simulink model "ClimaAdjmtOfTotFlowMgr"  (R2022b) (continued)
== system ClimaAdjmtOfTotFlowMgr == (continued)
  AirTFromHeatgEstimdForCCM_AirTFromHeatgEstimd/1 -> ClimaAdjmtOfTotFlowMgr_Runnable_sys/61(AirTFromHeatgEstimdForCCM_AirTFromHeatgEstimd_read)
  DrvrPfmncAlrmReq_DrvrPfmncAlrmReq/1 -> ClimaAdjmtOfTotFlowMgr_Runnable_sys/62(DrvrPfmncAlrmReq_DrvrPfmncAlrmReq_read)
  DrvrPfmncAlrmReqFromDHU_DrvrPfmncAlrmReqFromDHU/1 -> ClimaAdjmtOfTotFlowMgr_Runnable_sys/63(DrvrPfmncAlrmReqFromDHU_DrvrPfmncAlrmReqFromDHU_read)
  ClimaCfgForAirMtrlSnsrPrsnt1_ClimaCfgForAirMtrlSnsrPrsnt1/1 -> ClimaAdjmtOfTotFlowMgr_Runnable_sys/64(ClimaCfgForAirMtrlSnsrPrsnt1_ClimaCfgForAirMtrlSnsrPrsnt1_read)
  ClimaCfgForErev1_ClimaCfgForErev1/1 -> ClimaAdjmtOfTotFlowMgr_Runnable_sys/65(ClimaCfgForErev1_ClimaCfgForErev1_read)
  ClimaCfgReqVersionReplace_ClimaCfgReqVersionReplace/1 -> ClimaAdjmtOfTotFlowMgr_Runnable_sys/66(ClimaCfgReqVersionReplace_ClimaCfgReqVersionReplace_read)
  ClimaStStrtUpRe_ClimaStStrtUpRe/1 -> ClimaAdjmtOfTotFlowMgr_Runnable_sys/67(ClimaStStrtUpRe_ClimaStStrtUpRe_read)
  FlowRednForEngStby_FlowRednForEngStby/1 -> ClimaAdjmtOfTotFlowMgr_Runnable_sys/68(FlowRednForEngStby_FlowRednForEngStby_read)
  HmiCmptmtTSpForClima_HmiCmptmtTSpForClima/1 -> ClimaAdjmtOfTotFlowMgr_Runnable_sys/69(HmiCmptmtTSpForClima_HmiCmptmtTSpForClima_read)
  HvacAirTForRowFirstAtFlrLeRaw_HvacAirTForRowFirstAtFlrLeRaw/1 -> ClimaAdjmtOfTotFlowMgr_Runnable_sys/70(HvacAirTForRowFirstAtFlrLeRaw_HvacAirTForRowFirstAtFlrLeRaw_read)
  PrkgClimaOnReq_PrkgClimaOnReq/1 -> ClimaAdjmtOfTotFlowMgr_Runnable_sys/71(PrkgClimaOnReq_PrkgClimaOnReq_read)
  PrkgClimaOnReqRe_PrkgClimaOnReqRe/1 -> ClimaAdjmtOfTotFlowMgr_Runnable_sys/72(PrkgClimaOnReqRe_PrkgClimaOnReqRe_read)
  TModCtrlAtRowSec_TModCtrlAtRowSec/1 -> ClimaAdjmtOfTotFlowMgr_Runnable_sys/73(TModCtrlAtRowSec_TModCtrlAtRowSec_read)
  TModCtrlAtRowSec_TModCtrlAtRowSecLe/1 -> ClimaAdjmtOfTotFlowMgr_Runnable_sys/74(TModCtrlAtRowSec_TModCtrlAtRowSecLe_read)
  TModCtrlAtRowSec_TModCtrlAtRowSecRi/1 -> ClimaAdjmtOfTotFlowMgr_Runnable_sys/75(TModCtrlAtRowSec_TModCtrlAtRowSecRi_read)
  ClimaAdjmtOfTotFlowMgr_Runnable_sys/1(FlowDecDurgSpdIdleActvFlg_FlowDecDurgSpdIdleActvFlg_write) -> FlowDecDurgSpdIdleActvFlg_FlowDecDurgSpdIdleActvFlg/1
  ClimaAdjmtOfTotFlowMgr_Runnable_sys/2(FlowDecDurgSpdIdleActvFlgRe_FlowDecDurgSpdIdleActvFlgRe_write) -> FlowDecDurgSpdIdleActvFlgRe_FlowDecDurgSpdIdleActvFlgRe/1
  ClimaAdjmtOfTotFlowMgr_Runnable_sys/3(AirFlowLimForAirTLoActv_AirFlowLimForAirTLoActv_write) -> AirFlowLimForAirTLoActv_AirFlowLimForAirTLoActv/1
  ClimaAdjmtOfTotFlowMgr_Runnable_sys/4(ChgOfAirFlowForEngStb_ChgOfAirFlowForEngStb_write) -> ChgOfAirFlowForEngStb_ChgOfAirFlowForEngStb/1
  ClimaAdjmtOfTotFlowMgr_Runnable_sys/5(ChgOfFanLvlAut_ChgOfFanLvlAut_write) -> ChgOfFanLvlAut_ChgOfFanLvlAut/1
  ClimaAdjmtOfTotFlowMgr_Runnable_sys/6(ClimaAutCtrldFlowPredForDefrstAtStaby_ClimaAutCtrldFlowPredForDefrstAtStaby_write) -> ClimaAutCtrldFlowPredForDefrstAtStaby_ClimaAutCtrldFlowPredForDefrstAtStaby/1
  ClimaAdjmtOfTotFlowMgr_Runnable_sys/7(ClimaAutCtrldFlowPredForDefrstNoLim_ClimaAutCtrldFlowPredForDefrstNoLim_write) -> ClimaAutCtrldFlowPredForDefrstNoLim_ClimaAutCtrldFlowPredForDefrstNoLim/1
  ClimaAdjmtOfTotFlowMgr_Runnable_sys/8(ClimaAutCtrldFlowPredForRowFirstAtStaby_ClimaAutCtrldFlowPredForRowFirstAtStaby_write) -> ClimaAutCtrldFlowPredForRowFirstAtStaby_ClimaAutCtrldFlowPredForRowFirstAtStaby/1
  ClimaAdjmtOfTotFlowMgr_Runnable_sys/9(ClimaAutCtrldFlowPredForRowFirstNoLim_ClimaAutCtrldFlowPredForRowFirstNoLim_write) -> ClimaAutCtrldFlowPredForRowFirstNoLim_ClimaAutCtrldFlowPredForRowFirstNoLim/1
  ClimaAdjmtOfTotFlowMgr_Runnable_sys/10(ClimaAutCtrldFlowPredForRowSecAtStaby_ClimaAutCtrldFlowPredForRowSecAtStaby_write) -> ClimaAutCtrldFlowPredForRowSecAtStaby_ClimaAutCtrldFlowPredForRowSecAtStaby/1
  ClimaAdjmtOfTotFlowMgr_Runnable_sys/11(ClimaAutCtrldFlowPredForRowSecNoLim_ClimaAutCtrldFlowPredForRowSecNoLim_write) -> ClimaAutCtrldFlowPredForRowSecNoLim_ClimaAutCtrldFlowPredForRowSecNoLim/1
  ClimaAdjmtOfTotFlowMgr_Runnable_sys/12(ClimaAutCtrldFlowReqForDefrst_ClimaAutCtrldFlowReqForDefrst_write) -> ClimaAutCtrldFlowReqForDefrst_ClimaAutCtrldFlowReqForDefrst/1
  ClimaAdjmtOfTotFlowMgr_Runnable_sys/13(ClimaAutCtrldFlowReqForDefrst_ClimaAutCtrldFlowReqForDefrstLe_write) -> ClimaAutCtrldFlowReqForDefrst_ClimaAutCtrldFlowReqForDefrstLe/1
  ClimaAdjmtOfTotFlowMgr_Runnable_sys/14(ClimaAutCtrldFlowReqForDefrst_ClimaAutCtrldFlowReqForDefrstRi_write) -> ClimaAutCtrldFlowReqForDefrst_ClimaAutCtrldFlowReqForDefrstRi/1
  ClimaAdjmtOfTotFlowMgr_Runnable_sys/15(ClimaAutCtrldFlowReqForDefrstNoLim_ClimaAutCtrldFlowReqForDefrstNoLim_write) -> ClimaAutCtrldFlowReqForDefrstNoLim_ClimaAutCtrldFlowReqForDefrstNoLim/1
  ClimaAdjmtOfTotFlowMgr_Runnable_sys/16(ClimaAutCtrldTotFlowReqForRowFirst_ClimaAutCtrldTotFlowReqForRowFirst_write) -> ClimaAutCtrldTotFlowReqForRowFirst_ClimaAutCtrldTotFlowReqForRowFirst/1
  ClimaAdjmtOfTotFlowMgr_Runnable_sys/17(ClimaAutCtrldTotFlowReqForRowFirst_ClimaAutCtrldTotFlowReqForRowFirstLe_write) -> ClimaAutCtrldTotFlowReqForRowFirst_ClimaAutCtrldTotFlowReqForRowFirstLe/1
  ClimaAdjmtOfTotFlowMgr_Runnable_sys/18(ClimaAutCtrldTotFlowReqForRowFirst_ClimaAutCtrldTotFlowReqForRowFirstRi_write) -> ClimaAutCtrldTotFlowReqForRowFirst_ClimaAutCtrldTotFlowReqForRowFirstRi/1
  ClimaAdjmtOfTotFlowMgr_Runnable_sys/19(ClimaAutCtrldTotFlowReqForRowFirstNoLim_ClimaAutCtrldTotFlowReqForRowFirstNoLim_write) -> ClimaAutCtrldTotFlowReqForRowFirstNoLim_ClimaAutCtrldTotFlowReqForRowFirstNoLim/1
  ClimaAdjmtOfTotFlowMgr_Runnable_sys/20(ClimaAutCtrldTotFlowReqForRowSec_ClimaAutCtrldTotFlowReqForRowSec_write) -> ClimaAutCtrldTotFlowReqForRowSec_ClimaAutCtrldTotFlowReqForRowSec/1
  ClimaAdjmtOfTotFlowMgr_Runnable_sys/21(ClimaAutCtrldTotFlowReqForRowSec_ClimaAutCtrldTotFlowReqForRowSecLe_write) -> ClimaAutCtrldTotFlowReqForRowSec_ClimaAutCtrldTotFlowReqForRowSecLe/1
  ClimaAdjmtOfTotFlowMgr_Runnable_sys/22(ClimaAutCtrldTotFlowReqForRowSec_ClimaAutCtrldTotFlowReqForRowSecRi_write) -> ClimaAutCtrldTotFlowReqForRowSec_ClimaAutCtrldTotFlowReqForRowSecRi/1
  ClimaAdjmtOfTotFlowMgr_Runnable_sys/23(ClimaAutCtrldTotFlowReqForRowSecNoLim_ClimaAutCtrldTotFlowReqForRowSecNoLim_write) -> ClimaAutCtrldTotFlowReqForRowSecNoLim_ClimaAutCtrldTotFlowReqForRowSecNoLim/1
  ClimaAdjmtOfTotFlowMgr_Runnable_sys/24(FanLvlMaxActv_FanLvlMaxActv_write) -> FanLvlMaxActv_FanLvlMaxActv/1
  ClimaAdjmtOfTotFlowMgr_Runnable/1 -> ClimaAdjmtOfTotFlowMgr_Runnable_sys/Trigger

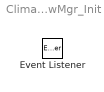
== system ClimaAdjmtOfTotFlowMgr/ClimaAdjmtOfTotFlowMgr_Init ==
  Event Listener : EventListener  EventName=reset

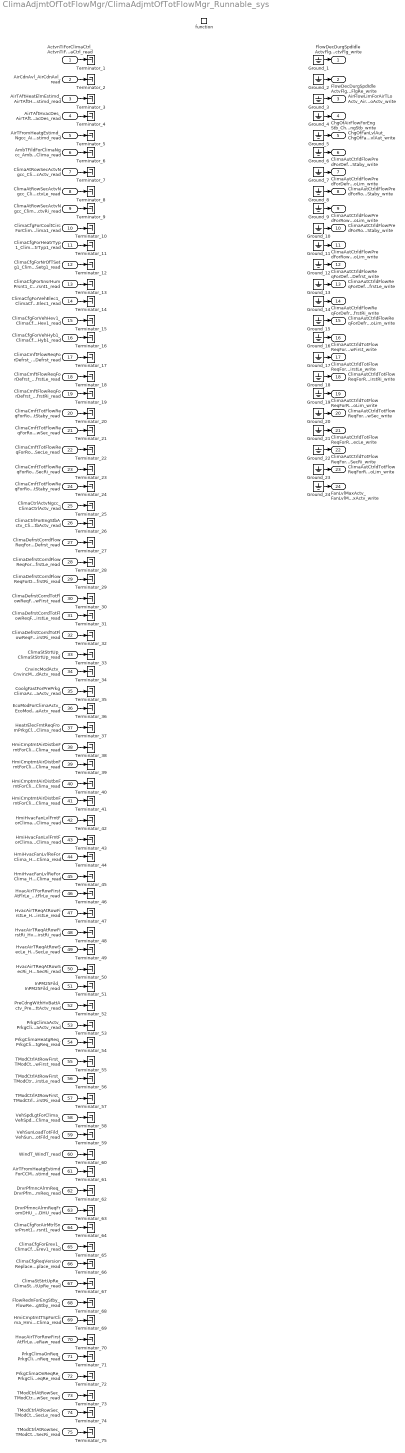
== system ClimaAdjmtOfTotFlowMgr/ClimaAdjmtOfTotFlowMgr_Runnable_sys ==
  ActvnTiForClimaCtrl_ActvnTiForClimaCtrl_read : Inport
  AirCdnAvl_AirCdnAvl_read : Inport  Port=2
  AirTAftHeatElmEstimd_AirTAftHeatElmEstimd_read : Inport  Port=3
  AirTAftHvacDes_AirTAftHvacDes_read : Inport  Port=4
  AirTFromHeatgEstimd_Ngcc_AirTFromHeatgEstimd_read : Inport  Port=5
  AmbTFildForClimaNgcc_AmbTFildForClima_read : Inport  Port=6
  ClimaAtRowSecActvNgcc_ClimaAtRowSecActv_read : Inport  Port=7
  ClimaAtRowSecActvNgcc_ClimaAtRowSecActvLe_read : Inport  Port=8
  ClimaAtRowSecActvNgcc_ClimaAtRowSecActvRi_read : Inport  Port=9
  ClimaCfgForCooltCircForClima1_ClimaCfgForCooltCircForClima1_read : Inport  Port=10
  ClimaCfgForHeatrTyp1_ClimaCfgForHeatrTyp1_read : Inport  Port=11
  ClimaCfgForNrOfTSetg1_ClimaCfgForNrOfTSetg1_read : Inport  Port=12
  ClimaCfgForSnsrHumPrsnt1_ClimaCfgForSnsrHumPrsnt1_read : Inport  Port=13
  ClimaCfgForVehElec1_ClimaCfgForVehElec1_read : Inport  Port=14
  ClimaCfgForVehHev1_ClimaCfgForVehHev1_read : Inport  Port=15
  ClimaCfgForVehHyb1_ClimaCfgForVehHyb1_read : Inport  Port=16
  ClimaCmftFlowReqForDefrst_ClimaCmftFlowReqForDefrst_read : Inport  Port=17
  ClimaCmftFlowReqForDefrst_ClimaCmftFlowReqForDefrstLe_read : Inport  Port=18
  ClimaCmftFlowReqForDefrst_ClimaCmftFlowReqForDefrstRi_read : Inport  Port=19
  ClimaCmftTotFlowReqForRowFirstAtStaby_ClimaCmftTotFlowReqForRowFirstAtStaby_read : Inport  Port=20
  ClimaCmftTotFlowReqForRowSec_ClimaCmftTotFlowReqForRowSec_read : Inport  Port=21
  ClimaCmftTotFlowReqForRowSec_ClimaCmftTotFlowReqForRowSecLe_read : Inport  Port=22
  ClimaCmftTotFlowReqForRowSec_ClimaCmftTotFlowReqForRowSecRi_read : Inport  Port=23
  ClimaCmftTotFlowReqForRowSecAtStaby_ClimaCmftTotFlowReqForRowSecAtStaby_read : Inport  Port=24
  ClimaCtrlActvNgcc_ClimaCtrlActv_read : Inport  Port=25
  ClimaCtrlForEngStbActv_ClimaCtrlForEngStbActv_read : Inport  Port=26
  ClimaDefrstCorrdFlowReqForDefrst_ClimaDefrstCorrdFlowReqForDefrst_read : Inport  Port=27
  ClimaDefrstCorrdFlowReqForDefrst_ClimaDefrstCorrdFlowReqForDefrstLe_read : Inport  Port=28
  ClimaDefrstCorrdFlowReqForDefrst_ClimaDefrstCorrdFlowReqForDefrstRi_read : Inport  Port=29
  ClimaDefrstCorrdTotFlowReqForRowFirst_ClimaDefrstCorrdTotFlowReqForRowFirst_read : Inport  Port=30
  ClimaDefrstCorrdTotFlowReqForRowFirst_ClimaDefrstCorrdTotFlowReqForRowFirstLe_read : Inport  Port=31
  ClimaDefrstCorrdTotFlowReqForRowFirst_ClimaDefrstCorrdTotFlowReqForRowFirstRi_read : Inport  Port=32
  ClimaStStrtUp_ClimaStStrtUp_read : Inport  Port=33
  CnvincModActv_CnvincModActv_read : Inport  Port=34
  CoolgFastForPrePrkgClimaActv_CoolgFastForPrePrkgClimaActv_read : Inport  Port=35
  EcoModForClimaActv_EcoModForClimaActv_read : Inport  Port=36
  HeatrElecFrntReqFromPrkgClima_HeatrElecFrntReqFromPrkgClima_read : Inport  Port=37
  HmiCmptmtAirDistbnFrntForClima_HmiCmptmtAirDistbnFrntForClima_read : Inport  Port=38
  HmiCmptmtAirDistbnFrntForClima_HmiCmptmtAirDistbnFrntRiForClima_read : Inport  Port=39
  HmiCmptmtAirDistbnFrntForClima_HmiCmptmtAirDistbnReForClima_read : Inport  Port=40
  HmiCmptmtAirDistbnFrntForClima_HmiCmptmtAirDistbnReRiForClima_read : Inport  Port=41
  HmiHvacFanLvlFrntForClima_HmiHvacFanLvlFrntForClima_read : Inport  Port=42
  HmiHvacFanLvlFrntForClima_HmiHvacFanLvlFrntRiForClima_read : Inport  Port=43
  HmiHvacFanLvlReForClima_HmiHvacFanLvlReForClima_read : Inport  Port=44
  HmiHvacFanLvlReForClima_HmiHvacFanLvlReRiForClima_read : Inport  Port=45
  HvacAirTForRowFirstAtFlrLe_HvacAirTForRowFirstAtFlrLe_HvacAirTForRowFirstAtFlrLe_read : Inport  Port=46
  HvacAirTReqAtRowFirstLe_HvacAirTReqAtRowFirstLe_read : Inport  Port=47
  HvacAirTReqAtRowFirstRi_HvacAirTReqAtRowFirstRi_read : Inport  Port=48
  HvacAirTReqAtRowSecLe_HvacAirTReqAtRowSecLe_read : Inport  Port=49
  HvacAirTReqAtRowSecRi_HvacAirTReqAtRowSecRi_read : Inport  Port=50
  InPM25Fild_InPM25Fild_read : Inport  Port=51
  PreCdngWithHvBattActv_PreCdngWithHvBattActv_read : Inport  Port=52
  PrkgClimaActv_PrkgClimaActv_read : Inport  Port=53
  PrkgClimaHeatgReq_PrkgClimaHeatgReq_read : Inport  Port=54
  TModCtrlAtRowFirst_TModCtrlAtRowFirst_read : Inport  Port=55
  TModCtrlAtRowFirst_TModCtrlAtRowFirstLe_read : Inport  Port=56
  TModCtrlAtRowFirst_TModCtrlAtRowFirstRi_read : Inport  Port=57
  VehSpdLgtForClima_VehSpdLgtForClima_read : Inport  Port=58
  VehSunLoadTotFild_VehSunLoadTotFild_read : Inport  Port=59
  WindT_WindT_read : Inport  Port=60
  AirTFromHeatgEstimdForCCM_AirTFromHeatgEstimd_read : Inport  Port=61
  DrvrPfmncAlrmReq_DrvrPfmncAlrmReq_read : Inport  Port=62
  DrvrPfmncAlrmReqFromDHU_DrvrPfmncAlrmReqFromDHU_read : Inport  Port=63
  ClimaCfgForAirMtrlSnsrPrsnt1_ClimaCfgForAirMtrlSnsrPrsnt1_read : Inport  Port=64
  ClimaCfgForErev1_ClimaCfgForErev1_read : Inport  Port=65
  ClimaCfgReqVersionReplace_ClimaCfgReqVersionReplace_read : Inport  Port=66
  ClimaStStrtUpRe_ClimaStStrtUpRe_read : Inport  Port=67
  FlowRednForEngStby_FlowRednForEngStby_read : Inport  Port=68
  HmiCmptmtTSpForClima_HmiCmptmtTSpForClima_read : Inport  Port=69
  HvacAirTForRowFirstAtFlrLeRaw_HvacAirTForRowFirstAtFlrLeRaw_read : Inport  Port=70
  PrkgClimaOnReq_PrkgClimaOnReq_read : Inport  Port=71
  PrkgClimaOnReqRe_PrkgClimaOnReqRe_read : Inport  Port=72
  TModCtrlAtRowSec_TModCtrlAtRowSec_read : Inport  Port=73
  TModCtrlAtRowSec_TModCtrlAtRowSecLe_read : Inport  Port=74
  TModCtrlAtRowSec_TModCtrlAtRowSecRi_read : Inport  Port=75
  function : TriggerPort  TriggerType=function-call  VariantControl=(inherit)  SampleTimeType=periodic  SampleTime=-1
  Ground_{1..24} (24 alike) : Ground
  Terminator_{1..75} (75 alike) : Terminator
  FlowDecDurgSpdIdleActvFlg_FlowDecDurgSpdIdleActvFlg_write : Outport
      settings: EnsureOutportIsVirtual=on
  FlowDecDurgSpdIdleActvFlgRe_FlowDecDurgSpdIdleActvFlgRe_write : Outport  Port=2
      settings: EnsureOutportIsVirtual=on
  AirFlowLimForAirTLoActv_AirFlowLimForAirTLoActv_write : Outport  Port=3
      settings: EnsureOutportIsVirtual=on
  ChgOfAirFlowForEngStb_ChgOfAirFlowForEngStb_write : Outport  Port=4
      settings: EnsureOutportIsVirtual=on
  ChgOfFanLvlAut_ChgOfFanLvlAut_write : Outport  Port=5
      settings: EnsureOutportIsVirtual=on
  ClimaAutCtrldFlowPredForDefrstAtStaby_ClimaAutCtrldFlowPredForDefrstAtStaby_write : Outport  Port=6
      settings: EnsureOutportIsVirtual=on
  ClimaAutCtrldFlowPredForDefrstNoLim_ClimaAutCtrldFlowPredForDefrstNoLim_write : Outport  Port=7
      settings: EnsureOutportIsVirtual=on
  ClimaAutCtrldFlowPredForRowFirstAtStaby_ClimaAutCtrldFlowPredForRowFirstAtStaby_write : Outport  Port=8
      settings: EnsureOutportIsVirtual=on
  ClimaAutCtrldFlowPredForRowFirstNoLim_ClimaAutCtrldFlowPredForRowFirstNoLim_write : Outport  Port=9
      settings: EnsureOutportIsVirtual=on
  ClimaAutCtrldFlowPredForRowSecAtStaby_ClimaAutCtrldFlowPredForRowSecAtStaby_write : Outport  Port=10
      settings: EnsureOutportIsVirtual=on
  ClimaAutCtrldFlowPredForRowSecNoLim_ClimaAutCtrldFlowPredForRowSecNoLim_write : Outport  Port=11
      settings: EnsureOutportIsVirtual=on
  ClimaAutCtrldFlowReqForDefrst_ClimaAutCtrldFlowReqForDefrst_write : Outport  Port=12
      settings: EnsureOutportIsVirtual=on
  ClimaAutCtrldFlowReqForDefrst_ClimaAutCtrldFlowReqForDefrstLe_write : Outport  Port=13
      settings: EnsureOutportIsVirtual=on
  ClimaAutCtrldFlowReqForDefrst_ClimaAutCtrldFlowReqForDefrstRi_write : Outport  Port=14
      settings: EnsureOutportIsVirtual=on
  ClimaAutCtrldFlowReqForDefrstNoLim_ClimaAutCtrldFlowReqForDefrstNoLim_write : Outport  Port=15
      settings: EnsureOutportIsVirtual=on
  ClimaAutCtrldTotFlowReqForRowFirst_ClimaAutCtrldTotFlowReqForRowFirst_write : Outport  Port=16
      settings: EnsureOutportIsVirtual=on
  ClimaAutCtrldTotFlowReqForRowFirst_ClimaAutCtrldTotFlowReqForRowFirstLe_write : Outport  Port=17
      settings: EnsureOutportIsVirtual=on
  ClimaAutCtrldTotFlowReqForRowFirst_ClimaAutCtrldTotFlowReqForRowFirstRi_write : Outport  Port=18
      settings: EnsureOutportIsVirtual=on
  ClimaAutCtrldTotFlowReqForRowFirstNoLim_ClimaAutCtrldTotFlowReqForRowFirstNoLim_write : Outport  Port=19
      settings: EnsureOutportIsVirtual=on
  ClimaAutCtrldTotFlowReqForRowSec_ClimaAutCtrldTotFlowReqForRowSec_write : Outport  Port=20
      settings: EnsureOutportIsVirtual=on
  ClimaAutCtrldTotFlowReqForRowSec_ClimaAutCtrldTotFlowReqForRowSecLe_write : Outport  Port=21
      settings: EnsureOutportIsVirtual=on
  ClimaAutCtrldTotFlowReqForRowSec_ClimaAutCtrldTotFlowReqForRowSecRi_write : Outport  Port=22
      settings: EnsureOutportIsVirtual=on
  ClimaAutCtrldTotFlowReqForRowSecNoLim_ClimaAutCtrldTotFlowReqForRowSecNoLim_write : Outport  Port=23
      settings: EnsureOutportIsVirtual=on
  FanLvlMaxActv_FanLvlMaxActv_write : Outport  Port=24
      settings: EnsureOutportIsVirtual=on
  wires:
  ActvnTiForClimaCtrl_ActvnTiForClimaCtrl_read/1 -> Terminator_1/1
  AirCdnAvl_AirCdnAvl_read/1 -> Terminator_2/1
  AirTAftHeatElmEstimd_AirTAftHeatElmEstimd_read/1 -> Terminator_3/1
  AirTAftHvacDes_AirTAftHvacDes_read/1 -> Terminator_4/1
  AirTFromHeatgEstimd_Ngcc_AirTFromHeatgEstimd_read/1 -> Terminator_5/1
  AmbTFildForClimaNgcc_AmbTFildForClima_read/1 -> Terminator_6/1
  ClimaAtRowSecActvNgcc_ClimaAtRowSecActv_read/1 -> Terminator_7/1
  ClimaAtRowSecActvNgcc_ClimaAtRowSecActvLe_read/1 -> Terminator_8/1
  ClimaAtRowSecActvNgcc_ClimaAtRowSecActvRi_read/1 -> Terminator_9/1
  ClimaCfgForCooltCircForClima1_ClimaCfgForCooltCircForClima1_read/1 -> Terminator_10/1
  ClimaCfgForHeatrTyp1_ClimaCfgForHeatrTyp1_read/1 -> Terminator_11/1
  ClimaCfgForNrOfTSetg1_ClimaCfgForNrOfTSetg1_read/1 -> Terminator_12/1
  ClimaCfgForSnsrHumPrsnt1_ClimaCfgForSnsrHumPrsnt1_read/1 -> Terminator_13/1
  ClimaCfgForVehElec1_ClimaCfgForVehElec1_read/1 -> Terminator_14/1
  ClimaCfgForVehHev1_ClimaCfgForVehHev1_read/1 -> Terminator_15/1
  ClimaCfgForVehHyb1_ClimaCfgForVehHyb1_read/1 -> Terminator_16/1
  ClimaCmftFlowReqForDefrst_ClimaCmftFlowReqForDefrst_read/1 -> Terminator_17/1
  ClimaCmftFlowReqForDefrst_ClimaCmftFlowReqForDefrstLe_read/1 -> Terminator_18/1
  ClimaCmftFlowReqForDefrst_ClimaCmftFlowReqForDefrstRi_read/1 -> Terminator_19/1
  ClimaCmftTotFlowReqForRowFirstAtStaby_ClimaCmftTotFlowReqForRowFirstAtStaby_read/1 -> Terminator_20/1
  ClimaCmftTotFlowReqForRowSec_ClimaCmftTotFlowReqForRowSec_read/1 -> Terminator_21/1
  ClimaCmftTotFlowReqForRowSec_ClimaCmftTotFlowReqForRowSecLe_read/1 -> Terminator_22/1
  ClimaCmftTotFlowReqForRowSec_ClimaCmftTotFlowReqForRowSecRi_read/1 -> Terminator_23/1
  ClimaCmftTotFlowReqForRowSecAtStaby_ClimaCmftTotFlowReqForRowSecAtStaby_read/1 -> Terminator_24/1
  ClimaCtrlActvNgcc_ClimaCtrlActv_read/1 -> Terminator_25/1
  ClimaCtrlForEngStbActv_ClimaCtrlForEngStbActv_read/1 -> Terminator_26/1
  ClimaDefrstCorrdFlowReqForDefrst_ClimaDefrstCorrdFlowReqForDefrst_read/1 -> Terminator_27/1
  ClimaDefrstCorrdFlowReqForDefrst_ClimaDefrstCorrdFlowReqForDefrstLe_read/1 -> Terminator_28/1
  ClimaDefrstCorrdFlowReqForDefrst_ClimaDefrstCorrdFlowReqForDefrstRi_read/1 -> Terminator_29/1
  ClimaDefrstCorrdTotFlowReqForRowFirst_ClimaDefrstCorrdTotFlowReqForRowFirst_read/1 -> Terminator_30/1
  ClimaDefrstCorrdTotFlowReqForRowFirst_ClimaDefrstCorrdTotFlowReqForRowFirstLe_read/1 -> Terminator_31/1
  ClimaDefrstCorrdTotFlowReqForRowFirst_ClimaDefrstCorrdTotFlowReqForRowFirstRi_read/1 -> Terminator_32/1
  ClimaStStrtUp_ClimaStStrtUp_read/1 -> Terminator_33/1
  CnvincModActv_CnvincModActv_read/1 -> Terminator_34/1
  CoolgFastForPrePrkgClimaActv_CoolgFastForPrePrkgClimaActv_read/1 -> Terminator_35/1
  EcoModForClimaActv_EcoModForClimaActv_read/1 -> Terminator_36/1
  HeatrElecFrntReqFromPrkgClima_HeatrElecFrntReqFromPrkgClima_read/1 -> Terminator_37/1
  HmiCmptmtAirDistbnFrntForClima_HmiCmptmtAirDistbnFrntForClima_read/1 -> Terminator_38/1
  HmiCmptmtAirDistbnFrntForClima_HmiCmptmtAirDistbnFrntRiForClima_read/1 -> Terminator_39/1
  HmiCmptmtAirDistbnFrntForClima_HmiCmptmtAirDistbnReForClima_read/1 -> Terminator_40/1
  HmiCmptmtAirDistbnFrntForClima_HmiCmptmtAirDistbnReRiForClima_read/1 -> Terminator_41/1
  HmiHvacFanLvlFrntForClima_HmiHvacFanLvlFrntForClima_read/1 -> Terminator_42/1
  HmiHvacFanLvlFrntForClima_HmiHvacFanLvlFrntRiForClima_read/1 -> Terminator_43/1
  HmiHvacFanLvlReForClima_HmiHvacFanLvlReForClima_read/1 -> Terminator_44/1
  HmiHvacFanLvlReForClima_HmiHvacFanLvlReRiForClima_read/1 -> Terminator_45/1
  HvacAirTForRowFirstAtFlrLe_HvacAirTForRowFirstAtFlrLe_HvacAirTForRowFirstAtFlrLe_read/1 -> Terminator_46/1
  HvacAirTReqAtRowFirstLe_HvacAirTReqAtRowFirstLe_read/1 -> Terminator_47/1
  HvacAirTReqAtRowFirstRi_HvacAirTReqAtRowFirstRi_read/1 -> Terminator_48/1
  HvacAirTReqAtRowSecLe_HvacAirTReqAtRowSecLe_read/1 -> Terminator_49/1
  HvacAirTReqAtRowSecRi_HvacAirTReqAtRowSecRi_read/1 -> Terminator_50/1
  InPM25Fild_InPM25Fild_read/1 -> Terminator_51/1
  PreCdngWithHvBattActv_PreCdngWithHvBattActv_read/1 -> Terminator_52/1
  PrkgClimaActv_PrkgClimaActv_read/1 -> Terminator_53/1
  PrkgClimaHeatgReq_PrkgClimaHeatgReq_read/1 -> Terminator_54/1
  TModCtrlAtRowFirst_TModCtrlAtRowFirst_read/1 -> Terminator_55/1
  TModCtrlAtRowFirst_TModCtrlAtRowFirstLe_read/1 -> Terminator_56/1
  TModCtrlAtRowFirst_TModCtrlAtRowFirstRi_read/1 -> Terminator_57/1
  VehSpdLgtForClima_VehSpdLgtForClima_read/1 -> Terminator_58/1
  VehSunLoadTotFild_VehSunLoadTotFild_read/1 -> Terminator_59/1
  WindT_WindT_read/1 -> Terminator_60/1
  AirTFromHeatgEstimdForCCM_AirTFromHeatgEstimd_read/1 -> Terminator_61/1
  DrvrPfmncAlrmReq_DrvrPfmncAlrmReq_read/1 -> Terminator_62/1
  DrvrPfmncAlrmReqFromDHU_DrvrPfmncAlrmReqFromDHU_read/1 -> Terminator_63/1
  ClimaCfgForAirMtrlSnsrPrsnt1_ClimaCfgForAirMtrlSnsrPrsnt1_read/1 -> Terminator_64/1
  ClimaCfgForErev1_ClimaCfgForErev1_read/1 -> Terminator_65/1
  ClimaCfgReqVersionReplace_ClimaCfgReqVersionReplace_read/1 -> Terminator_66/1
  ClimaStStrtUpRe_ClimaStStrtUpRe_read/1 -> Terminator_67/1
  FlowRednForEngStby_FlowRednForEngStby_read/1 -> Terminator_68/1
  HmiCmptmtTSpForClima_HmiCmptmtTSpForClima_read/1 -> Terminator_69/1
  HvacAirTForRowFirstAtFlrLeRaw_HvacAirTForRowFirstAtFlrLeRaw_read/1 -> Terminator_70/1
  PrkgClimaOnReq_PrkgClimaOnReq_read/1 -> Terminator_71/1
  PrkgClimaOnReqRe_PrkgClimaOnReqRe_read/1 -> Terminator_72/1
  TModCtrlAtRowSec_TModCtrlAtRowSec_read/1 -> Terminator_73/1
  TModCtrlAtRowSec_TModCtrlAtRowSecLe_read/1 -> Terminator_74/1
  TModCtrlAtRowSec_TModCtrlAtRowSecRi_read/1 -> Terminator_75/1
  Ground_1/1 -> FlowDecDurgSpdIdleActvFlg_FlowDecDurgSpdIdleActvFlg_write/1
  Ground_2/1 -> FlowDecDurgSpdIdleActvFlgRe_FlowDecDurgSpdIdleActvFlgRe_write/1
  Ground_3/1 -> AirFlowLimForAirTLoActv_AirFlowLimForAirTLoActv_write/1
  Ground_4/1 -> ChgOfAirFlowForEngStb_ChgOfAirFlowForEngStb_write/1
  Ground_5/1 -> ChgOfFanLvlAut_ChgOfFanLvlAut_write/1
  Ground_6/1 -> ClimaAutCtrldFlowPredForDefrstAtStaby_ClimaAutCtrldFlowPredForDefrstAtStaby_write/1
  Ground_7/1 -> ClimaAutCtrldFlowPredForDefrstNoLim_ClimaAutCtrldFlowPredForDefrstNoLim_write/1
  Ground_8/1 -> ClimaAutCtrldFlowPredForRowFirstAtStaby_ClimaAutCtrldFlowPredForRowFirstAtStaby_write/1
  Ground_9/1 -> ClimaAutCtrldFlowPredForRowFirstNoLim_ClimaAutCtrldFlowPredForRowFirstNoLim_write/1
  Ground_10/1 -> ClimaAutCtrldFlowPredForRowSecAtStaby_ClimaAutCtrldFlowPredForRowSecAtStaby_write/1
  Ground_11/1 -> ClimaAutCtrldFlowPredForRowSecNoLim_ClimaAutCtrldFlowPredForRowSecNoLim_write/1
  Ground_12/1 -> ClimaAutCtrldFlowReqForDefrst_ClimaAutCtrldFlowReqForDefrst_write/1
  Ground_13/1 -> ClimaAutCtrldFlowReqForDefrst_ClimaAutCtrldFlowReqForDefrstLe_write/1
  Ground_14/1 -> ClimaAutCtrldFlowReqForDefrst_ClimaAutCtrldFlowReqForDefrstRi_write/1
  Ground_15/1 -> ClimaAutCtrldFlowReqForDefrstNoLim_ClimaAutCtrldFlowReqForDefrstNoLim_write/1
  Ground_16/1 -> ClimaAutCtrldTotFlowReqForRowFirst_ClimaAutCtrldTotFlowReqForRowFirst_write/1
  Ground_17/1 -> ClimaAutCtrldTotFlowReqForRowFirst_ClimaAutCtrldTotFlowReqForRowFirstLe_write/1
  Ground_18/1 -> ClimaAutCtrldTotFlowReqForRowFirst_ClimaAutCtrldTotFlowReqForRowFirstRi_write/1
  Ground_19/1 -> ClimaAutCtrldTotFlowReqForRowFirstNoLim_ClimaAutCtrldTotFlowReqForRowFirstNoLim_write/1
  Ground_20/1 -> ClimaAutCtrldTotFlowReqForRowSec_ClimaAutCtrldTotFlowReqForRowSec_write/1
  Ground_21/1 -> ClimaAutCtrldTotFlowReqForRowSec_ClimaAutCtrldTotFlowReqForRowSecLe_write/1
  Ground_22/1 -> ClimaAutCtrldTotFlowReqForRowSec_ClimaAutCtrldTotFlowReqForRowSecRi_write/1
  Ground_23/1 -> ClimaAutCtrldTotFlowReqForRowSecNoLim_ClimaAutCtrldTotFlowReqForRowSecNoLim_write/1
  Ground_24/1 -> FanLvlMaxActv_FanLvlMaxActv_write/1
